simulink model "TosAeb"  (R2021a)
settings: Solver=FixedStepDiscrete  StartTime=0.0  StopTime=inf  FixedStep=0.01  RelTol=1e-3
undefined symbols (not defined in the file or in its folder): ACCModSts, AccSts1, AEB_ObjectMovingOrientation, AEB_ObjectMovingState, CN_BTCActive, CN_CurrentGearR, CN_CurrentGearR_DHT, CN_PTCActive, CN_VDCActive, CN_VSC_EspOff, CN_VSC_FCW_AEB_Error, CN_VSC_FCW_AEB_NoDispay, CN_VSC_FCW_AEB_PerfmDgdtn, CN_VSC_FCW_AEB_TempUnAvlbl, CN_VSC_VehStandstill, CNaccelAvoidMax, CNaccelAvoidMin, CNbrakePedalPress, CNcomfortStrLeftAccelMax, CNcomfortStrLeftAccelMin, CNcomfortStrRightAccelMax, CNcomfortStrRightAccelMin, CNDrvDoorOpen, CNDrvModeEco, CNDrvModeNorm, CNDrvModeOther, CNDrvModeSport, CNDrvSBRreminder, CNNull, CNobjInvalidID, CNPassDoorOpen, CNPassSBRreminder, CNPEBSAccelNegInf, CNPEBSAccelNull, CNPEBSTimeInf, CNPEBSTimeNull, CNSTOPMAX, CNSTOPNULL, CNsubjWidth, CNSwtOFF, CNSwtON, CNTTCMAX, CNTTCNULL, CNVehConfigAEBCycNone, CNVehConfigAEBNone, CNVehConfigAEBPedNone, CNVehConfigFCWNone, CNVehConfigJANone, CollisionSeverity, FALSE, FCWSnvty, JACycObjCalcInfoDefaultParams, JAPedObjCalcInfoDefaultParams, JAVehObjCalcInfoDefaultParams, latCycObjCalcInfoDefaultParams, latPedObjCalcInfoDefaultParams, longObjCalcInfoDefaultParams, ModSts, movingStatus, ObjectType ... (+1112)
libraries: simulink x3031, AEBSFunctionLib x50, Ta_Library x33, AEBSStateMachineLib x23, Mylibrary x12, simulink_extras x7, ObjSelect_lib x3, dspstat3 x2
(part 84 of 102)

== system TosAeb/FcwAebJa_Step_sys/ALG_AEBSystem/ALG_AEBController/AEB_LongTgtInfoCalc/LongObjCalcInfoCalc/AEBS_LongCollisionAssesApp/LongCollisionAvoidAssess/HighLvlBrkCalc/HighLvlBrkCalc4Warning/accelAvoidHighLvlBrkSituation1/accelAvoidLowLvlBrkCalc2/Situation1/Case1 ==
  axAbsObj : Inport
  vx : Inport  Port=2
  dxEgoBrake : Inport  Port=3
  Action Port : ActionPort
  Divide : Product  Inputs=*/
  Gain : Gain  Gain=2
  Math Function : Math  Operator=square  SignedPower=on  SaturateOnIntegerOverflow=on
  Subtract : Sum  Inputs=+-
  accelAvoid : Outport
  wires:
  vx/1 -> Math Function/1
  Math Function/1 -> Divide/1
  Gain/1 -> Divide/2
  dxEgoBrake/1 -> Gain/1
  Divide/1 -> Subtract/2
  axAbsObj/1 -> Subtract/1
  Subtract/1 -> accelAvoid/1

== system TosAeb/FcwAebJa_Step_sys/ALG_AEBSystem/ALG_AEBController/AEB_LongTgtInfoCalc/LongObjCalcInfoCalc/AEBS_LongCollisionAssesApp/LongCollisionAvoidAssess/HighLvlBrkCalc/HighLvlBrkCalc4Warning/accelAvoidHighLvlBrkSituation1/accelAvoidLowLvlBrkCalc2/Situation1/Case2 ==
  note: vEgo^2
      | aAvoid = ----------------------------------
      |                   vObj^2
      |                  ---------  - 2(dRel - dScure)
      |                   aObj
  subjVel : Inport
  vxAbsObj : Inport  Port=2
  axAbsObj : Inport  Port=3
  dxEgoBrake : Inport  Port=4
  Action Port : ActionPort
  Divide : Product  Inputs=*/
  Divide1 : Product  Inputs=*/
  Gain : Gain  Gain=2
  Math Function : Math  Operator=square  SignedPower=on  SaturateOnIntegerOverflow=on
  Math Function1 : Math  Operator=square  SignedPower=on  SaturateOnIntegerOverflow=on
  Subtract : Sum  Inputs=+-
  accelAvoid : Outport
  wires:
  vxAbsObj/1 -> Math Function/1
  Math Function/1 -> Divide/1
  axAbsObj/1 -> Divide/2
  Divide/1 -> Subtract/1
  dxEgoBrake/1 -> Gain/1
  Gain/1 -> Subtract/2
  subjVel/1 -> Math Function1/1
  Subtract/1 -> Divide1/2
  Math Function1/1 -> Divide1/1
  Divide1/1 -> accelAvoid/1

== system TosAeb/FcwAebJa_Step_sys/ALG_AEBSystem/ALG_AEBController/AEB_LongTgtInfoCalc/LongObjCalcInfoCalc/AEBS_LongCollisionAssesApp/LongCollisionAvoidAssess/HighLvlBrkCalc/HighLvlBrkCalc4Warning/accelAvoidHighLvlBrkSituation1/accelAvoidLowLvlBrkCalc2/Situation1/collisionFirstFlgCalc ==
  note: Calculate Ego and Obj Collision Time and
      |  Determine if the Collision Time is less than the object stop time
  dxEgoBrake : Inport
  vx : Inport  Port=2
  tObjStop : Inport  Port=3
  Divide : Product  Inputs=*/
  Gain : Gain  Gain=-1
  Gain1 : Gain  Gain=2
  Relational Operator : RelationalOperator  Operator=<  OutDataTypeStr=boolean  RndMeth=Simplest
  Saturation : Saturate  UpperLimit=CNTTCMAX  LowerLimit=CNTTCNULL
      settings: AttributesFormatString=|
      | UpperLimit:%<UpperLimit>
      | LowerLimit:%<LowerLimit>
  collisionFirstFlg : Outport
  wires:
  Divide/1 -> Gain1/1
  dxEgoBrake/1 -> Divide/1
  vx/1 -> Gain/1
  Gain/1 -> Divide/2
  Gain1/1 -> Saturation/1
  tObjStop/1 -> Relational Operator/2
  Relational Operator/1 -> collisionFirstFlg/1
  Saturation/1 -> Relational Operator/1

== system TosAeb/FcwAebJa_Step_sys/ALG_AEBSystem/ALG_AEBController/AEB_LongTgtInfoCalc/LongObjCalcInfoCalc/AEBS_LongCollisionAssesApp/LongCollisionAvoidAssess/HighLvlBrkCalc/HighLvlBrkCalc4Warning/accelAvoidHighLvlBrkSituation2/accelAvoidHighLvlBrkCalc/If Action Subsystem1/accelAvoidLowLvlBrkCalc ==
  vx : Inport
  axAbsObj : Inport  Port=2
  vxAbsObj : Inport  Port=3
  subjVel : Inport  Port=4
  tObjStop : Inport  Port=5
  dxEgoBrake : Inport  Port=6
  AccelAvoidSituationJudge : Stateflow chart  TreatAsAtomicUnit=on
  Bus Creator : BusCreator  Inputs=6
  Bus Selector : BusSelector  OutputSignals=vx,axAbsObj
  Bus Selector1 : BusSelector  OutputSignals=dxEgoBrake,vx,tObjStop,subjVel,vxAbsObj,axAbsObj
  Bus Selector2 : BusSelector  OutputSignals=axAbsObj,vx,dxEgoBrake
  Bus Selector3 : BusSelector  OutputSignals=subjVel,vxAbsObj,axAbsObj,dxEgoBrake
  Merge : Merge  Inputs=4
  Situation1 : Subsystem  TreatAsAtomicUnit=on
  Situation2 : Subsystem  TreatAsAtomicUnit=on
  Situation3 : Subsystem  TreatAsAtomicUnit=on
  Situation4 : Subsystem  TreatAsAtomicUnit=on
  accelAvoidLowLvlBrkLimit : Subsystem
  accelAvoidLowLvlBrk : Outport
  wires:
  accelAvoidLowLvlBrkLimit/1(accelAvoidLowLvlBrk) -> accelAvoidLowLvlBrk/1
  Merge/1 -> accelAvoidLowLvlBrkLimit/1(accelAvoidLowLvlBrkNoLimit)
  Bus Selector3/4 -> Situation3/4(dxEgoBrake)  "<dxEgoBrake>"
  Bus Selector3/3 -> Situation3/3(axAbsObj)  "<axAbsObj>"
  Bus Selector3/2 -> Situation3/2(vxAbsObj)  "<vxAbsObj>"
  Bus Selector3/1 -> Situation3/1(subjVel)  "<subjVel>"
  Bus Selector2/1 -> Situation2/1(axAbsObj)  "<axAbsObj>"
  Bus Selector2/2 -> Situation2/2(vx)  "<vx>"
  Bus Selector2/3 -> Situation2/3(dxEgoBrake)  "<dxEgoBrake>"
  Bus Selector/2 -> AccelAvoidSituationJudge/2(axAbsObj)  "<axAbsObj>"
  Bus Selector/1 -> AccelAvoidSituationJudge/1(vx)  "<vx>"
  Bus Creator/1 -> Bus Selector/1, Bus Selector1/1, Bus Selector2/1, Bus Selector3/1
  tObjStop/1 -> Bus Creator/5  "tObjStop"
  axAbsObj/1 -> Bus Creator/2  "axAbsObj"
  vx/1 -> Bus Creator/1  "vx"
  dxEgoBrake/1 -> Bus Creator/6  "dxEgoBrake"
  subjVel/1 -> Bus Creator/4  "subjVel"
  vxAbsObj/1 -> Bus Creator/3  "vxAbsObj"
  [Situation4] -> Situation4/Trigger
  [Situation3] -> Situation3/Trigger
  [Situation2] -> Situation2/Trigger
  AccelAvoidSituationJudge/4(Situation4()) -> [Situation4]
  AccelAvoidSituationJudge/3(Situation3()) -> [Situation3]
  AccelAvoidSituationJudge/2(Situation2()) -> [Situation2]
  Situation4/1(accelAvoidLowLvlBrk) -> Merge/4
  Situation3/1(accelAvoidLowLvlBrk) -> Merge/3
  Situation2/1(accelAvoidLowLvlBrk) -> Merge/2
  Situation1/1(accelAvoidLowLvlBrk) -> Merge/1
  AccelAvoidSituationJudge/1(Situation1()) -> Situation1/Trigger
  Bus Selector1/1 -> Situation1/1(dxEgoBrake)  "<dxEgoBrake>"
  Bus Selector1/2 -> Situation1/2(vx)  "<vx>"
  Bus Selector1/3 -> Situation1/3(tObjStop)  "<tObjStop>"
  Bus Selector1/4 -> Situation1/4(subjVel)  "<subjVel>"
  Bus Selector1/5 -> Situation1/5(vxAbsObj)  "<vxAbsObj>"
  Bus Selector1/6 -> Situation1/6(axAbsObj)  "<axAbsObj>"

== system TosAeb/FcwAebJa_Step_sys/ALG_AEBSystem/ALG_AEBController/AEB_LongTgtInfoCalc/LongObjCalcInfoCalc/AEBS_LongCollisionAssesApp/LongCollisionAvoidAssess/HighLvlBrkCalc/HighLvlBrkCalc4Warning/accelAvoidHighLvlBrkSituation2/accelAvoidHighLvlBrkCalc/If Action Subsystem1/accelAvoidLowLvlBrkCalc1 ==
  vx : Inport
  axAbsObj : Inport  Port=2
  vxAbsObj : Inport  Port=3
  subjVel : Inport  Port=4
  tObjStop : Inport  Port=5
  dxEgoBrake : Inport  Port=6
  AccelAvoidSituationJudge : Stateflow chart  TreatAsAtomicUnit=on
  Bus Creator : BusCreator  Inputs=6
  Bus Selector : BusSelector  OutputSignals=vxRelTargetObjCalc,axAbsTargetObjCalc
  Bus Selector1 : BusSelector  OutputSignals=dxEgoBrake,vxRelTargetObjCalc,tObjStop,subjVel,vxAbsTargetObjCalc,axAbsTargetObjCalc
  Bus Selector2 : BusSelector  OutputSignals=axAbsTargetObjCalc,vxRelTargetObjCalc,dxEgoBrake
  Bus Selector3 : BusSelector  OutputSignals=subjVel,vxAbsTargetObjCalc,axAbsTargetObjCalc,dxEgoBrake
  Merge : Merge  Inputs=4
  Situation1 : Subsystem  TreatAsAtomicUnit=on
  Situation2 : Subsystem  TreatAsAtomicUnit=on
  Situation3 : Subsystem  TreatAsAtomicUnit=on
  Situation4 : Subsystem  TreatAsAtomicUnit=on
  accelAvoidLowLvlBrkLimit : Subsystem
  accelAvoidLowLvlBrk : Outport
  wires:
  Bus Selector1/6 -> Situation1/6(axAbsObj)  "<axAbsTargetObjCalc>"
  Bus Selector1/5 -> Situation1/5(vxAbsObj)  "<vxAbsTargetObjCalc>"
  Bus Selector1/4 -> Situation1/4(subjVel)  "<subjVel>"
  Bus Selector1/3 -> Situation1/3(tObjStop)  "<tObjStop>"
  Bus Selector1/2 -> Situation1/2(vx)  "<vxRelTargetObjCalc>"
  Bus Selector1/1 -> Situation1/1(dxEgoBrake)  "<dxEgoBrake>"
  AccelAvoidSituationJudge/1(Situation1()) -> Situation1/Trigger
  Situation1/1(accelAvoidLowLvlBrk) -> Merge/1
  Situation2/1(accelAvoidLowLvlBrk) -> Merge/2
  Situation3/1(accelAvoidLowLvlBrk) -> Merge/3
  Situation4/1(accelAvoidLowLvlBrk) -> Merge/4
  AccelAvoidSituationJudge/2(Situation2()) -> [Situation2]
  AccelAvoidSituationJudge/3(Situation3()) -> [Situation3]
  AccelAvoidSituationJudge/4(Situation4()) -> [Situation4]
  [Situation2] -> Situation2/Trigger
  [Situation3] -> Situation3/Trigger
  [Situation4] -> Situation4/Trigger
  vxAbsObj/1 -> Bus Creator/3  "vxAbsTargetObjCalc"
  subjVel/1 -> Bus Creator/4  "subjVel"
  dxEgoBrake/1 -> Bus Creator/6  "dxEgoBrake"
  vx/1 -> Bus Creator/1  "vxRelTargetObjCalc"
  axAbsObj/1 -> Bus Creator/2  "axAbsTargetObjCalc"
  tObjStop/1 -> Bus Creator/5  "tObjStop"
  Bus Creator/1 -> Bus Selector3/1, Bus Selector2/1, Bus Selector1/1, Bus Selector/1
  Bus Selector/1 -> AccelAvoidSituationJudge/1(vxRelTargetObjCalc)  "<vxRelTargetObjCalc>"
  Bus Selector/2 -> AccelAvoidSituationJudge/2(axAbsTargetObjCalc)  "<axAbsTargetObjCalc>"
  Bus Selector2/3 -> Situation2/3(dxEgoBrake)  "<dxEgoBrake>"
  Bus Selector2/2 -> Situation2/2(vx)  "<vxRelTargetObjCalc>"
  Bus Selector2/1 -> Situation2/1(axAbsObj)  "<axAbsTargetObjCalc>"
  Bus Selector3/1 -> Situation3/1(subjVel)  "<subjVel>"
  Bus Selector3/2 -> Situation3/2(vxAbsObj)  "<vxAbsTargetObjCalc>"
  Bus Selector3/3 -> Situation3/3(axAbsObj)  "<axAbsTargetObjCalc>"
  Bus Selector3/4 -> Situation3/4(dxEgoBrake)  "<dxEgoBrake>"
  Merge/1 -> accelAvoidLowLvlBrkLimit/1(accelAvoidLowLvlBrkNoLimit)
  accelAvoidLowLvlBrkLimit/1(accelAvoidLowLvlBrk) -> accelAvoidLowLvlBrk/1

== system TosAeb/FcwAebJa_Step_sys/ALG_AEBSystem/ALG_AEBController/AEB_LongTgtInfoCalc/LongObjCalcInfoCalc/AEBS_LongCollisionAssesApp/LongCollisionAvoidAssess/HighLvlBrkCalc/HighLvlBrkCalc4Warning/accelAvoidHighLvlBrkSituation2/accelAvoidHighLvlBrkCalc/tObjStopCalc/StopTimeCalc ==
  velRel : Inport
  accelRel : Inport  Port=2
  tStopFuncCalc : TriggerPort  TriggerType=function-call  VariantControl=Variant
  Abs : Abs
  Abs1 : Abs
  Divide : Product  Inputs=*/
  tStop : Outport
  wires:
  velRel/1 -> Abs/1
  accelRel/1 -> Abs1/1
  Abs/1 -> Divide/1
  Abs1/1 -> Divide/2
  Divide/1 -> tStop/1

== system TosAeb/FcwAebJa_Step_sys/ALG_AEBSystem/ALG_AEBController/AEB_LongTgtInfoCalc/LongObjCalcInfoCalc/AEBS_LongCollisionAssesApp/LongCollisionAvoidAssess/HighLvlBrkCalc/HighLvlBrkCalc4Warning/accelAvoidHighLvlBrkSituation3/If Action Subsystem1/DistReactionCalc/If Action Subsystem ==
  Action Port : ActionPort  ActionPortLabel=if(u1)
  Constant : Constant  Value=CNNull
  dxObjReaction : Outport
  wires:
  Constant/1 -> dxObjReaction/1

== system TosAeb/FcwAebJa_Step_sys/ALG_AEBSystem/ALG_AEBController/AEB_LongTgtInfoCalc/LongObjCalcInfoCalc/AEBS_LongCollisionAssesApp/LongCollisionAvoidAssess/HighLvlBrkCalc/HighLvlBrkCalc4Warning/accelAvoidHighLvlBrkSituation3/If Action Subsystem1/DistReactionCalc/If Action Subsystem1 ==
  vxAbsObj : Inport
  axAbsObj : Inport  Port=2
  Action Port : ActionPort  ActionPortLabel=else
  Divide : Product  Inputs=*/
  Gain : Gain  Gain=-2
  Math Function : Math  Operator=square  SignedPower=on  SaturateOnIntegerOverflow=on
  dxObjReaction : Outport
  wires:
  Divide/1 -> dxObjReaction/1
  Gain/1 -> Divide/2
  axAbsObj/1 -> Gain/1
  Math Function/1 -> Divide/1
  vxAbsObj/1 -> Math Function/1

== system TosAeb/FcwAebJa_Step_sys/ALG_AEBSystem/ALG_AEBController/AEB_LongTgtInfoCalc/LongObjCalcInfoCalc/AEBS_LongCollisionAssesApp/LongCollisionAvoidAssess/HighLvlBrkCalc/HighLvlBrkCalc4Warning/accelAvoidHighLvlBrkSituation3/If Action Subsystem1/accelAvoidHighLvlBrkCalc/MovingSateCalcAfterReactionTime ==
  note: For Situation 3,after driver reaction time ,the object has been stopped.
      | Ego vehicle is keeping moving.
  subjLongAccel : Inport
  tReaction : Inport  Port=2
  subjVel : Inport  Port=3
  Add2 : Sum
  Constant : Constant  Value=CNNull
  Product3 : Product
  Subtract : Sum  Inputs=+-
  vxRelTargetPredict : Outport
  axAbsTargetPredict : Outport  Port=2
  vxAbsTargetPredict : Outport  Port=3
  subjVelPredict : Outport  Port=4
  tObjStopPredict : Outport  Port=5
  wires:
  Add2/1 -> subjVelPredict/1, Subtract/2
  Subtract/1 -> vxRelTargetPredict/1
  Constant/1 -> axAbsTargetPredict/1, tObjStopPredict/1, vxAbsTargetPredict/1, Subtract/1
  tReaction/1 -> Product3/2
  subjLongAccel/1 -> Product3/1
  Product3/1 -> Add2/1
  subjVel/1 -> Add2/2

== system TosAeb/FcwAebJa_Step_sys/ALG_AEBSystem/ALG_AEBController/AEB_LongTgtInfoCalc/LongObjCalcInfoCalc/AEBS_LongCollisionAssesApp/LongCollisionAvoidAssess/HighLvlBrkCalc/HighLvlBrkCalc4Warning/accelAvoidHighLvlBrkSituation3/If Action Subsystem1/accelAvoidHighLvlBrkCalc/accelAvoidLowLvlBrkCalc ==
  vx : Inport
  axAbsObj : Inport  Port=2
  vxAbsObj : Inport  Port=3
  subjVel : Inport  Port=4
  tObjStop : Inport  Port=5
  dxEgoBrake : Inport  Port=6
  AccelAvoidSituationJudge : Stateflow chart  TreatAsAtomicUnit=on
  Bus Creator : BusCreator  Inputs=6
  Bus Selector : BusSelector  OutputSignals=vx,axAbsObj
  Bus Selector1 : BusSelector  OutputSignals=dxEgoBrake,vx,tObjStop,subjVel,vxAbsObj,axAbsObj
  Bus Selector2 : BusSelector  OutputSignals=axAbsObj,vx,dxEgoBrake
  Bus Selector3 : BusSelector  OutputSignals=subjVel,vxAbsObj,axAbsObj,dxEgoBrake
  Merge : Merge  Inputs=4
  Situation1 : Subsystem  TreatAsAtomicUnit=on
  Situation2 : Subsystem  TreatAsAtomicUnit=on
  Situation3 : Subsystem  TreatAsAtomicUnit=on
  Situation4 : Subsystem  TreatAsAtomicUnit=on
  accelAvoidLowLvlBrkLimit : Subsystem
  accelAvoidLowLvlBrk : Outport
  wires:
  accelAvoidLowLvlBrkLimit/1(accelAvoidLowLvlBrk) -> accelAvoidLowLvlBrk/1
  Merge/1 -> accelAvoidLowLvlBrkLimit/1(accelAvoidLowLvlBrkNoLimit)
  Bus Selector3/4 -> Situation3/4(dxEgoBrake)  "<dxEgoBrake>"
  Bus Selector3/3 -> Situation3/3(axAbsObj)  "<axAbsObj>"
  Bus Selector3/2 -> Situation3/2(vxAbsObj)  "<vxAbsObj>"
  Bus Selector3/1 -> Situation3/1(subjVel)  "<subjVel>"
  Bus Selector2/1 -> Situation2/1(axAbsObj)  "<axAbsObj>"
  Bus Selector2/2 -> Situation2/2(vx)  "<vx>"
  Bus Selector2/3 -> Situation2/3(dxEgoBrake)  "<dxEgoBrake>"
  Bus Selector/2 -> AccelAvoidSituationJudge/2(axAbsObj)  "<axAbsObj>"
  Bus Selector/1 -> AccelAvoidSituationJudge/1(vx)  "<vx>"
  Bus Creator/1 -> Bus Selector/1, Bus Selector1/1, Bus Selector2/1, Bus Selector3/1
  tObjStop/1 -> Bus Creator/5  "tObjStop"
  axAbsObj/1 -> Bus Creator/2  "axAbsObj"
  vx/1 -> Bus Creator/1  "vx"
  dxEgoBrake/1 -> Bus Creator/6  "dxEgoBrake"
  subjVel/1 -> Bus Creator/4  "subjVel"
  vxAbsObj/1 -> Bus Creator/3  "vxAbsObj"
  [Situation4] -> Situation4/Trigger
  [Situation3] -> Situation3/Trigger
  [Situation2] -> Situation2/Trigger
  AccelAvoidSituationJudge/4(Situation4()) -> [Situation4]
  AccelAvoidSituationJudge/3(Situation3()) -> [Situation3]
  AccelAvoidSituationJudge/2(Situation2()) -> [Situation2]
  Situation4/1(accelAvoidLowLvlBrk) -> Merge/4
  Situation3/1(accelAvoidLowLvlBrk) -> Merge/3
  Situation2/1(accelAvoidLowLvlBrk) -> Merge/2
  Situation1/1(accelAvoidLowLvlBrk) -> Merge/1
  AccelAvoidSituationJudge/1(Situation1()) -> Situation1/Trigger
  Bus Selector1/1 -> Situation1/1(dxEgoBrake)  "<dxEgoBrake>"
  Bus Selector1/2 -> Situation1/2(vx)  "<vx>"
  Bus Selector1/3 -> Situation1/3(tObjStop)  "<tObjStop>"
  Bus Selector1/4 -> Situation1/4(subjVel)  "<subjVel>"
  Bus Selector1/5 -> Situation1/5(vxAbsObj)  "<vxAbsObj>"
  Bus Selector1/6 -> Situation1/6(axAbsObj)  "<axAbsObj>"

== system TosAeb/FcwAebJa_Step_sys/ALG_AEBSystem/ALG_AEBController/AEB_LongTgtInfoCalc/LongObjCalcInfoCalc/AEBS_LongCollisionAssesApp/LongCollisionAvoidAssess/HighLvlBrkCalc/HighLvlBrkCalc4Warning/accelAvoidHighLvlBrkSituation3/If Action Subsystem1/accelAvoidHighLvlBrkCalc/accelAvoidLowLvlBrkCalc1 ==
  vx : Inport
  axAbsObj : Inport  Port=2
  vxAbsObj : Inport  Port=3
  subjVel : Inport  Port=4
  tObjStop : Inport  Port=5
  dxEgoBrake : Inport  Port=6
  AccelAvoidSituationJudge : Stateflow chart  TreatAsAtomicUnit=on
  Bus Creator : BusCreator  Inputs=6
  Bus Selector : BusSelector  OutputSignals=vxRelTargetObjCalc,axAbsTargetObjCalc
  Bus Selector1 : BusSelector  OutputSignals=dxEgoBrake,vxRelTargetObjCalc,tObjStop,subjVel,vxAbsTargetObjCalc,axAbsTargetObjCalc
  Bus Selector2 : BusSelector  OutputSignals=axAbsTargetObjCalc,vxRelTargetObjCalc,dxEgoBrake
  Bus Selector3 : BusSelector  OutputSignals=subjVel,vxAbsTargetObjCalc,axAbsTargetObjCalc,dxEgoBrake
  Merge : Merge  Inputs=4
  Situation1 : Subsystem  TreatAsAtomicUnit=on
  Situation2 : Subsystem  TreatAsAtomicUnit=on
  Situation3 : Subsystem  TreatAsAtomicUnit=on
  Situation4 : Subsystem  TreatAsAtomicUnit=on
  accelAvoidLowLvlBrkLimit : Subsystem
  accelAvoidLowLvlBrk : Outport
  wires:
  Bus Selector1/6 -> Situation1/6(axAbsObj)  "<axAbsTargetObjCalc>"
  Bus Selector1/5 -> Situation1/5(vxAbsObj)  "<vxAbsTargetObjCalc>"
  Bus Selector1/4 -> Situation1/4(subjVel)  "<subjVel>"
  Bus Selector1/3 -> Situation1/3(tObjStop)  "<tObjStop>"
  Bus Selector1/2 -> Situation1/2(vx)  "<vxRelTargetObjCalc>"
  Bus Selector1/1 -> Situation1/1(dxEgoBrake)  "<dxEgoBrake>"
  AccelAvoidSituationJudge/1(Situation1()) -> Situation1/Trigger
  Situation1/1(accelAvoidLowLvlBrk) -> Merge/1
  Situation2/1(accelAvoidLowLvlBrk) -> Merge/2
  Situation3/1(accelAvoidLowLvlBrk) -> Merge/3
  Situation4/1(accelAvoidLowLvlBrk) -> Merge/4
  AccelAvoidSituationJudge/2(Situation2()) -> [Situation2]
  AccelAvoidSituationJudge/3(Situation3()) -> [Situation3]
  AccelAvoidSituationJudge/4(Situation4()) -> [Situation4]
  [Situation2] -> Situation2/Trigger
  [Situation3] -> Situation3/Trigger
  [Situation4] -> Situation4/Trigger
  vxAbsObj/1 -> Bus Creator/3  "vxAbsTargetObjCalc"
  subjVel/1 -> Bus Creator/4  "subjVel"
  dxEgoBrake/1 -> Bus Creator/6  "dxEgoBrake"
  vx/1 -> Bus Creator/1  "vxRelTargetObjCalc"
  axAbsObj/1 -> Bus Creator/2  "axAbsTargetObjCalc"
  tObjStop/1 -> Bus Creator/5  "tObjStop"
  Bus Creator/1 -> Bus Selector3/1, Bus Selector2/1, Bus Selector1/1, Bus Selector/1
  Bus Selector/1 -> AccelAvoidSituationJudge/1(vxRelTargetObjCalc)  "<vxRelTargetObjCalc>"
  Bus Selector/2 -> AccelAvoidSituationJudge/2(axAbsTargetObjCalc)  "<axAbsTargetObjCalc>"
  Bus Selector2/3 -> Situation2/3(dxEgoBrake)  "<dxEgoBrake>"
  Bus Selector2/2 -> Situation2/2(vx)  "<vxRelTargetObjCalc>"
  Bus Selector2/1 -> Situation2/1(axAbsObj)  "<axAbsTargetObjCalc>"
  Bus Selector3/1 -> Situation3/1(subjVel)  "<subjVel>"
  Bus Selector3/2 -> Situation3/2(vxAbsObj)  "<vxAbsTargetObjCalc>"
  Bus Selector3/3 -> Situation3/3(axAbsObj)  "<axAbsTargetObjCalc>"
  Bus Selector3/4 -> Situation3/4(dxEgoBrake)  "<dxEgoBrake>"
  Merge/1 -> accelAvoidLowLvlBrkLimit/1(accelAvoidLowLvlBrkNoLimit)
  accelAvoidLowLvlBrkLimit/1(accelAvoidLowLvlBrk) -> accelAvoidLowLvlBrk/1

== system TosAeb/FcwAebJa_Step_sys/ALG_AEBSystem/ALG_AEBController/AEB_LongTgtInfoCalc/LongObjCalcInfoCalc/AEBS_LongCollisionAssesApp/LongCollisionAvoidAssess/HighLvlBrkCalc/HighLvlBrkCalc4Warning/accelAvoidHighLvlBrkSituation5/If Action Subsystem1/If Action Subsystem1/If Action Subsystem2 ==
  Action Port : ActionPort  ActionPortLabel=if(u1)
  Constant : Constant  Value=CNNull
  dxObjReaction : Outport
  wires:
  Constant/1 -> dxObjReaction/1

== system TosAeb/FcwAebJa_Step_sys/ALG_AEBSystem/ALG_AEBController/AEB_LongTgtInfoCalc/LongObjCalcInfoCalc/AEBS_LongCollisionAssesApp/LongCollisionAvoidAssess/HighLvlBrkCalc/HighLvlBrkCalc4Warning/accelAvoidHighLvlBrkSituation5/If Action Subsystem1/If Action Subsystem1/If Action Subsystem3 ==
  vxAbsObj : Inport
  axAbsObj : Inport  Port=2
  Action Port : ActionPort  ActionPortLabel=else
  Divide : Product  Inputs=*/
  Gain : Gain  Gain=-2
  Math Function : Math  Operator=square  SignedPower=on  SaturateOnIntegerOverflow=on
  dxObjReaction : Outport
  wires:
  Divide/1 -> dxObjReaction/1
  Gain/1 -> Divide/2
  axAbsObj/1 -> Gain/1
  Math Function/1 -> Divide/1
  vxAbsObj/1 -> Math Function/1

== system TosAeb/FcwAebJa_Step_sys/ALG_AEBSystem/ALG_AEBController/AEB_LongTgtInfoCalc/LongObjCalcInfoCalc/AEBS_LongCollisionAssesApp/collisionSeverityHighCalc/collisionSeverityHighCalc1/CollisionAssessHighCalcVersion3/CollisionAssessHighCalc1/CalcBrakePhase1/tEgoStopCalc/tStopCalc ==
  accelAbs : Inport
  velAbs : Inport  Port=2
  StopTimeFlow : Stateflow chart  TreatAsAtomicUnit=on
  tStop : Outport
  wires:
  velAbs/1 -> StopTimeFlow/2(velRel)  "velAbs"
  accelAbs/1 -> StopTimeFlow/1(accelRel)  "accelAbs"
  StopTimeFlow/1(tStop) -> tStop/1  "tStop"

== system TosAeb/FcwAebJa_Step_sys/ALG_AEBSystem/ALG_AEBController/AEB_LongTgtInfoCalc/LongObjCalcInfoCalc/AEBS_LongCollisionAssesApp/collisionSeverityHighCalc/collisionSeverityHighCalc1/CollisionAssessHighCalcVersion3/CollisionAssessHighCalc1/CalcBrakePhase1/tObjStopCalc/tStopCalc ==
  accelAbs : Inport
  velAbs : Inport  Port=2
  StopTimeFlow : Stateflow chart  TreatAsAtomicUnit=on
  tStop : Outport
  wires:
  velAbs/1 -> StopTimeFlow/2(velRel)  "velAbs"
  accelAbs/1 -> StopTimeFlow/1(accelRel)  "accelAbs"
  StopTimeFlow/1(tStop) -> tStop/1  "tStop"

== system TosAeb/FcwAebJa_Step_sys/ALG_AEBSystem/ALG_AEBController/AEB_LongTgtInfoCalc/LongObjCalcInfoCalc/AEBS_LongCollisionAssesApp/collisionSeverityHighCalc/collisionSeverityHighCalc1/CollisionAssessHighCalcVersion3/CollisionAssessHighCalc1/CalcBrakePhase1/timeEgovDeltaCalc/timeEgovDelata ==
  accelAbs : Inport
  velAbs : Inport  Port=2
  StopTimeFlow : Stateflow chart  TreatAsAtomicUnit=on
  tStop : Outport
  wires:
  velAbs/1 -> StopTimeFlow/2(velRel)  "velAbs"
  accelAbs/1 -> StopTimeFlow/1(accelRel)  "accelAbs"
  StopTimeFlow/1(tStop) -> tStop/1  "tStop"

== system TosAeb/FcwAebJa_Step_sys/ALG_AEBSystem/ALG_AEBController/AEB_LongTgtInfoCalc/LongObjCalcInfoCalc/AEBS_LongCollisionAssesApp/collisionSeverityHighCalc/collisionSeverityHighCalc1/CollisionAssessHighCalcVersion3/CollisionAssessHighCalc1/CalcBrakePhase1/timeEgovDeltaCalc/velMaxReudceFiltCalc ==
  v0Ego : Inport
  velMaxReudce : Inport  Port=2
  MinMax : MinMax  Inputs=2
  velMaxReudceFilt : Outport
  wires:
  MinMax/1 -> velMaxReudceFilt/1
  velMaxReudce/1 -> MinMax/2
  v0Ego/1 -> MinMax/1

== system TosAeb/FcwAebJa_Step_sys/ALG_AEBSystem/ALG_AEBController/AEB_LongTgtInfoCalc/LongObjCalcInfoCalc/AEBS_LongCollisionAssesApp/collisionSeverityHighCalc/collisionSeverityHighCalc1/CollisionAssessHighCalcVersion3/CollisionAssessHighCalc1/CalcBrakePhase2/BrkSysRuntimeFhase2/If Action Subsystem ==
  a0Ego : Inport
  accelMaxDec : Inport  Port=2
  accelGradient : Inport  Port=3
  Action Port : ActionPort  ActionPortLabel=if(u1)
  Divide : Product  Inputs=*/
  Subtract : Sum  Inputs=+-
  tTime : Outport
  wires:
  accelMaxDec/1 -> Subtract/1
  a0Ego/1 -> Subtract/2
  Subtract/1 -> Divide/1
  accelGradient/1 -> Divide/2
  Divide/1 -> tTime/1

== system TosAeb/FcwAebJa_Step_sys/ALG_AEBSystem/ALG_AEBController/AEB_LongTgtInfoCalc/LongObjCalcInfoCalc/AEBS_LongCollisionAssesApp/collisionSeverityHighCalc/collisionSeverityHighCalc1/CollisionAssessHighCalcVersion3/CollisionAssessHighCalc1/CalcBrakePhase2/BrkSysRuntimeFhase2/If Action Subsystem1 ==
  Action Port : ActionPort  ActionPortLabel=else
  Constant : Constant  Value=CNNull
  tTime : Outport
  wires:
  Constant/1 -> tTime/1

== system TosAeb/FcwAebJa_Step_sys/ALG_AEBSystem/ALG_AEBController/AEB_LongTgtInfoCalc/LongObjCalcInfoCalc/AEBS_LongCollisionAssesApp/collisionSeverityHighCalc/collisionSeverityHighCalc1/CollisionAssessHighCalcVersion3/CollisionAssessHighCalc1/CalcBrakePhase2/BrkSysRuntimeFhase2/accelEgoLessAccelmaxReduce ==
  accelMaxDec : Inport
  a0Ego : Inport  Port=2
  Relational Operator : RelationalOperator  Operator=<  OutDataTypeStr=boolean  RndMeth=Simplest
  Flg : Outport
  wires:
  Relational Operator/1 -> Flg/1
  accelMaxDec/1 -> Relational Operator/1
  a0Ego/1 -> Relational Operator/2

== system TosAeb/FcwAebJa_Step_sys/ALG_AEBSystem/ALG_AEBController/AEB_LongTgtInfoCalc/LongObjCalcInfoCalc/AEBS_LongCollisionAssesApp/collisionSeverityHighCalc/collisionSeverityHighCalc1/CollisionAssessHighCalcVersion3/CollisionAssessHighCalc1/CalcBrakePhase2/tEgoStopCalc/tStopCalc ==
  accelAbs : Inport
  velAbs : Inport  Port=2
  StopTimeFlow : Stateflow chart  TreatAsAtomicUnit=on
  tStop : Outport
  wires:
  velAbs/1 -> StopTimeFlow/2(velRel)  "velAbs"
  accelAbs/1 -> StopTimeFlow/1(accelRel)  "accelAbs"
  StopTimeFlow/1(tStop) -> tStop/1  "tStop"

== system TosAeb/FcwAebJa_Step_sys/ALG_AEBSystem/ALG_AEBController/AEB_LongTgtInfoCalc/LongObjCalcInfoCalc/AEBS_LongCollisionAssesApp/collisionSeverityHighCalc/collisionSeverityHighCalc1/CollisionAssessHighCalcVersion3/CollisionAssessHighCalc1/CalcBrakePhase2/tObjStopCalc/tStopCalc ==
  accelAbs : Inport
  velAbs : Inport  Port=2
  StopTimeFlow : Stateflow chart  TreatAsAtomicUnit=on
  tStop : Outport
  wires:
  velAbs/1 -> StopTimeFlow/2(velRel)  "velAbs"
  accelAbs/1 -> StopTimeFlow/1(accelRel)  "accelAbs"
  StopTimeFlow/1(tStop) -> tStop/1  "tStop"

== system TosAeb/FcwAebJa_Step_sys/ALG_AEBSystem/ALG_AEBController/AEB_LongTgtInfoCalc/LongObjCalcInfoCalc/AEBS_LongCollisionAssesApp/collisionSeverityHighCalc/collisionSeverityHighCalc1/CollisionAssessHighCalcVersion3/CollisionAssessHighCalc1/CalcBrakePhase2/timeEgovDeltaCalc/timeEgovDelata ==
  accelAbs : Inport
  velAbs : Inport  Port=2
  StopTimeFlow : Stateflow chart  TreatAsAtomicUnit=on
  tStop : Outport
  wires:
  velAbs/1 -> StopTimeFlow/2(velRel)  "velAbs"
  accelAbs/1 -> StopTimeFlow/1(accelRel)  "accelAbs"
  StopTimeFlow/1(tStop) -> tStop/1  "tStop"

== system TosAeb/FcwAebJa_Step_sys/ALG_AEBSystem/ALG_AEBController/AEB_LongTgtInfoCalc/LongObjCalcInfoCalc/AEBS_LongCollisionAssesApp/collisionSeverityHighCalc/collisionSeverityHighCalc1/CollisionAssessHighCalcVersion3/CollisionAssessHighCalc1/CalcBrakePhase2/timeEgovDeltaCalc/velMaxReudceFiltCalc ==
  v0Ego : Inport
  velMaxReudce : Inport  Port=2
  MinMax : MinMax  Inputs=2
  velMaxReudceFilt : Outport
  wires:
  MinMax/1 -> velMaxReudceFilt/1
  velMaxReudce/1 -> MinMax/2
  v0Ego/1 -> MinMax/1

== system TosAeb/FcwAebJa_Step_sys/ALG_AEBSystem/ALG_AEBController/AEB_LongTgtInfoCalc/LongObjCalcInfoCalc/AEBS_LongCollisionAssesApp/collisionSeverityHighCalc/collisionSeverityHighCalc1/CollisionAssessHighCalcVersion3/CollisionAssessHighCalc1/CalcBrakePhase3/BrkSysRuntime/Subsystem ==
  a0Obj : Inport
  accelMaxDec : Inport  Port=2
  Abs : Abs
  Compare To Constant : simulink/Logic and Bit Operations/Compare To Constant  relop=<  const=1e-6  OutDataTypeStr=boolean
  Subtract : Sum  Inputs=+-
  Flg : Outport
  wires:
  Abs/1 -> Compare To Constant/1
  accelMaxDec/1 -> Subtract/2
  a0Obj/1 -> Subtract/1
  Subtract/1 -> Abs/1
  Compare To Constant/1 -> Flg/1

== system TosAeb/FcwAebJa_Step_sys/ALG_AEBSystem/ALG_AEBController/AEB_LongTgtInfoCalc/LongObjCalcInfoCalc/AEBS_LongCollisionAssesApp/collisionSeverityHighCalc/collisionSeverityHighCalc1/CollisionAssessHighCalcVersion3/CollisionAssessHighCalc1/CalcBrakePhase3/BrkSysRuntime/a0ObjGreatSituation ==
  vtEgo : Inport
  accelMaxDec : Inport  Port=2
  v0Obj : Inport  Port=3
  a0Obj : Inport  Port=4
  Action Port : ActionPort  ActionPortLabel=else
  If : If  IfExpression=u1
  Merge : Merge
  Subsystem : Subsystem
  vtEgoGreatSituation : Subsystem  TreatAsAtomicUnit=on
  vtEgoLessSituation : Subsystem  TreatAsAtomicUnit=on
  tTime : Outport
  wires:
  [vtEgoGreat] -> vtEgoGreatSituation/Action
  If/2 -> [vtEgoGreat]
  Subsystem/1(Flg) -> If/1
  vtEgo/1 -> vtEgoLessSituation/2(vtEgo), Subsystem/1(vtEgo)
  v0Obj/1 -> vtEgoLessSituation/1(v0Obj), Subsystem/2(v0Obj)
  If/1 -> vtEgoLessSituation/Action
  vtEgoLessSituation/1(tTime) -> Merge/1
  vtEgoGreatSituation/1(tTime) -> Merge/2
  Merge/1 -> tTime/1
  accelMaxDec/1 -> vtEgoLessSituation/3(accelMaxDec)
  a0Obj/1 -> vtEgoLessSituation/4(a0Obj)

== system TosAeb/FcwAebJa_Step_sys/ALG_AEBSystem/ALG_AEBController/AEB_LongTgtInfoCalc/LongObjCalcInfoCalc/AEBS_LongCollisionAssesApp/collisionSeverityHighCalc/collisionSeverityHighCalc1/CollisionAssessHighCalcVersion3/CollisionAssessHighCalc1/CalcBrakePhase3/BrkSysRuntime/a0ObjLessSituation ==
  vtEgo : Inport
  accelMaxDec : Inport  Port=2
  Action Port : ActionPort  ActionPortLabel=if(u1)
  Divide : Product  Inputs=*/
  Unary Minus : UnaryMinus
  tTime : Outport
  wires:
  vtEgo/1 -> Unary Minus/1
  Unary Minus/1 -> Divide/1
  accelMaxDec/1 -> Divide/2
  Divide/1 -> tTime/1

== system TosAeb/FcwAebJa_Step_sys/ALG_AEBSystem/ALG_AEBController/AEB_LongTgtInfoCalc/LongObjCalcInfoCalc/AEBS_LongCollisionAssesApp/collisionSeverityHighCalc/collisionSeverityHighCalc1/CollisionAssessHighCalcVersion3/CollisionAssessHighCalc1/CalcBrakePhase3/tEgoStopCalc/tStopCalc ==
  accelAbs : Inport
  velAbs : Inport  Port=2
  StopTimeFlow : Stateflow chart  TreatAsAtomicUnit=on
  tStop : Outport
  wires:
  velAbs/1 -> StopTimeFlow/2(velRel)  "velAbs"
  accelAbs/1 -> StopTimeFlow/1(accelRel)  "accelAbs"
  StopTimeFlow/1(tStop) -> tStop/1  "tStop"

== system TosAeb/FcwAebJa_Step_sys/ALG_AEBSystem/ALG_AEBController/AEB_LongTgtInfoCalc/LongObjCalcInfoCalc/AEBS_LongCollisionAssesApp/collisionSeverityHighCalc/collisionSeverityHighCalc1/CollisionAssessHighCalcVersion3/CollisionAssessHighCalc1/CalcBrakePhase3/tObjStopCalc/tStopCalc ==
  accelAbs : Inport
  velAbs : Inport  Port=2
  StopTimeFlow : Stateflow chart  TreatAsAtomicUnit=on
  tStop : Outport
  wires:
  velAbs/1 -> StopTimeFlow/2(velRel)  "velAbs"
  accelAbs/1 -> StopTimeFlow/1(accelRel)  "accelAbs"
  StopTimeFlow/1(tStop) -> tStop/1  "tStop"

== system TosAeb/FcwAebJa_Step_sys/ALG_AEBSystem/ALG_AEBController/AEB_LongTgtInfoCalc/LongObjCalcInfoCalc/AEBS_LongCollisionAssesApp/collisionSeverityHighCalc/collisionSeverityHighCalc1/CollisionAssessHighCalcVersion3/CollisionAssessHighCalc1/CalcBrakePhase3/timeEgovDeltaCalc/timeEgovDelata ==
  accelAbs : Inport
  velAbs : Inport  Port=2
  StopTimeFlow : Stateflow chart  TreatAsAtomicUnit=on
  tStop : Outport
  wires:
  velAbs/1 -> StopTimeFlow/2(velRel)  "velAbs"
  accelAbs/1 -> StopTimeFlow/1(accelRel)  "accelAbs"
  StopTimeFlow/1(tStop) -> tStop/1  "tStop"

== system TosAeb/FcwAebJa_Step_sys/ALG_AEBSystem/ALG_AEBController/AEB_LongTgtInfoCalc/LongObjCalcInfoCalc/AEBS_LongCollisionAssesApp/collisionSeverityHighCalc/collisionSeverityHighCalc1/CollisionAssessHighCalcVersion3/CollisionAssessHighCalc1/CalcBrakePhase3/timeEgovDeltaCalc/velMaxReudceFiltCalc ==
  v0Ego : Inport
  velMaxReudce : Inport  Port=2
  MinMax : MinMax  Inputs=2
  velMaxReudceFilt : Outport
  wires:
  MinMax/1 -> velMaxReudceFilt/1
  velMaxReudce/1 -> MinMax/2
  v0Ego/1 -> MinMax/1

== system TosAeb/FcwAebJa_Step_sys/ALG_AEBSystem/ALG_AEBController/AEB_LongTgtInfoCalc/LongObjCalcInfoCalc/AEBS_LongCollisionAssesApp/collisionSeverityHighCalc/collisionSeverityHighCalc1/CollisionAssessHighCalcVersion3/CollisionAssessHighCalc2/CalcBrakePhase1/tEgoStopCalc/tStopCalc ==
  accelAbs : Inport
  velAbs : Inport  Port=2
  StopTimeFlow : Stateflow chart  TreatAsAtomicUnit=on
  tStop : Outport
  wires:
  velAbs/1 -> StopTimeFlow/2(velRel)  "velAbs"
  accelAbs/1 -> StopTimeFlow/1(accelRel)  "accelAbs"
  StopTimeFlow/1(tStop) -> tStop/1  "tStop"

== system TosAeb/FcwAebJa_Step_sys/ALG_AEBSystem/ALG_AEBController/AEB_LongTgtInfoCalc/LongObjCalcInfoCalc/AEBS_LongCollisionAssesApp/collisionSeverityHighCalc/collisionSeverityHighCalc1/CollisionAssessHighCalcVersion3/CollisionAssessHighCalc2/CalcBrakePhase1/tObjStopCalc/tStopCalc ==
  accelAbs : Inport
  velAbs : Inport  Port=2
  StopTimeFlow : Stateflow chart  TreatAsAtomicUnit=on
  tStop : Outport
  wires:
  velAbs/1 -> StopTimeFlow/2(velRel)  "velAbs"
  accelAbs/1 -> StopTimeFlow/1(accelRel)  "accelAbs"
  StopTimeFlow/1(tStop) -> tStop/1  "tStop"

== system TosAeb/FcwAebJa_Step_sys/ALG_AEBSystem/ALG_AEBController/AEB_LongTgtInfoCalc/LongObjCalcInfoCalc/AEBS_LongCollisionAssesApp/collisionSeverityHighCalc/collisionSeverityHighCalc1/CollisionAssessHighCalcVersion3/CollisionAssessHighCalc2/CalcBrakePhase1/timeEgovDelataCalc/timeEgovDelata ==
  accelAbs : Inport
  velAbs : Inport  Port=2
  StopTimeFlow : Stateflow chart  TreatAsAtomicUnit=on
  tStop : Outport
  wires:
  velAbs/1 -> StopTimeFlow/2(velRel)  "velAbs"
  accelAbs/1 -> StopTimeFlow/1(accelRel)  "accelAbs"
  StopTimeFlow/1(tStop) -> tStop/1  "tStop"

== system TosAeb/FcwAebJa_Step_sys/ALG_AEBSystem/ALG_AEBController/AEB_LongTgtInfoCalc/LongObjCalcInfoCalc/AEBS_LongCollisionAssesApp/collisionSeverityHighCalc/collisionSeverityHighCalc1/CollisionAssessHighCalcVersion3/CollisionAssessHighCalc2/CalcBrakePhase1/timeEgovDelataCalc/velMaxReudceFiltCalc ==
  v0Ego : Inport
  velMaxReudce : Inport  Port=2
  MinMax : MinMax  Inputs=2
  velMaxReudceFilt : Outport
  wires:
  MinMax/1 -> velMaxReudceFilt/1
  velMaxReudce/1 -> MinMax/2
  v0Ego/1 -> MinMax/1

== system TosAeb/FcwAebJa_Step_sys/ALG_AEBSystem/ALG_AEBController/AEB_LongTgtInfoCalc/LongObjCalcInfoCalc/AEBS_LongCollisionAssesApp/collisionSeverityHighCalc/collisionSeverityHighCalc1/CollisionAssessHighCalcVersion3/CollisionAssessHighCalc2/CalcBrakePhase2/BrkSysRuntimeFhase2/If Action Subsystem ==
  a0Ego : Inport
  accelMaxDec : Inport  Port=2
  accelGradient : Inport  Port=3
  Action Port : ActionPort
  Divide : Product  Inputs=*/
  Subtract : Sum  Inputs=+-
  tTime : Outport
  wires:
  accelMaxDec/1 -> Subtract/1
  a0Ego/1 -> Subtract/2
  Subtract/1 -> Divide/1
  accelGradient/1 -> Divide/2
  Divide/1 -> tTime/1

== system TosAeb/FcwAebJa_Step_sys/ALG_AEBSystem/ALG_AEBController/AEB_LongTgtInfoCalc/LongObjCalcInfoCalc/AEBS_LongCollisionAssesApp/collisionSeverityHighCalc/collisionSeverityHighCalc1/CollisionAssessHighCalcVersion3/CollisionAssessHighCalc2/CalcBrakePhase2/BrkSysRuntimeFhase2/If Action Subsystem1 ==
  Action Port : ActionPort
  Constant : Constant  Value=0
  tTime : Outport
  wires:
  Constant/1 -> tTime/1

== system TosAeb/FcwAebJa_Step_sys/ALG_AEBSystem/ALG_AEBController/AEB_LongTgtInfoCalc/LongObjCalcInfoCalc/AEBS_LongCollisionAssesApp/collisionSeverityHighCalc/collisionSeverityHighCalc1/CollisionAssessHighCalcVersion3/CollisionAssessHighCalc2/CalcBrakePhase2/BrkSysRuntimeFhase2/accelEgoLessAccelmaxReduce ==
  accelMaxDec : Inport
  a0Ego : Inport  Port=2
  Relational Operator : RelationalOperator  Operator=<  OutDataTypeStr=boolean  RndMeth=Simplest
  Flg : Outport
  wires:
  Relational Operator/1 -> Flg/1
  accelMaxDec/1 -> Relational Operator/1
  a0Ego/1 -> Relational Operator/2

== system TosAeb/FcwAebJa_Step_sys/ALG_AEBSystem/ALG_AEBController/AEB_LongTgtInfoCalc/LongObjCalcInfoCalc/AEBS_LongCollisionAssesApp/collisionSeverityHighCalc/collisionSeverityHighCalc1/CollisionAssessHighCalcVersion3/CollisionAssessHighCalc2/CalcBrakePhase2/tEgoStopCalc/tStopCalc ==
  accelAbs : Inport
  velAbs : Inport  Port=2
  StopTimeFlow : Stateflow chart  TreatAsAtomicUnit=on
  tStop : Outport
  wires:
  velAbs/1 -> StopTimeFlow/2(velRel)  "velAbs"
  accelAbs/1 -> StopTimeFlow/1(accelRel)  "accelAbs"
  StopTimeFlow/1(tStop) -> tStop/1  "tStop"

== system TosAeb/FcwAebJa_Step_sys/ALG_AEBSystem/ALG_AEBController/AEB_LongTgtInfoCalc/LongObjCalcInfoCalc/AEBS_LongCollisionAssesApp/collisionSeverityHighCalc/collisionSeverityHighCalc1/CollisionAssessHighCalcVersion3/CollisionAssessHighCalc2/CalcBrakePhase2/tObjStopCalc/tStopCalc ==
  accelAbs : Inport
  velAbs : Inport  Port=2
  StopTimeFlow : Stateflow chart  TreatAsAtomicUnit=on
  tStop : Outport
  wires:
  velAbs/1 -> StopTimeFlow/2(velRel)  "velAbs"
  accelAbs/1 -> StopTimeFlow/1(accelRel)  "accelAbs"
  StopTimeFlow/1(tStop) -> tStop/1  "tStop"

== system TosAeb/FcwAebJa_Step_sys/ALG_AEBSystem/ALG_AEBController/AEB_LongTgtInfoCalc/LongObjCalcInfoCalc/AEBS_LongCollisionAssesApp/collisionSeverityHighCalc/collisionSeverityHighCalc1/CollisionAssessHighCalcVersion3/CollisionAssessHighCalc2/CalcBrakePhase2/timeEgovDelataCalc/timeEgovDelata ==
  accelAbs : Inport
  velAbs : Inport  Port=2
  StopTimeFlow : Stateflow chart  TreatAsAtomicUnit=on
  tStop : Outport
  wires:
  velAbs/1 -> StopTimeFlow/2(velRel)  "velAbs"
  accelAbs/1 -> StopTimeFlow/1(accelRel)  "accelAbs"
  StopTimeFlow/1(tStop) -> tStop/1  "tStop"

== system TosAeb/FcwAebJa_Step_sys/ALG_AEBSystem/ALG_AEBController/AEB_LongTgtInfoCalc/LongObjCalcInfoCalc/AEBS_LongCollisionAssesApp/collisionSeverityHighCalc/collisionSeverityHighCalc1/CollisionAssessHighCalcVersion3/CollisionAssessHighCalc2/CalcBrakePhase2/timeEgovDelataCalc/velMaxReudceFiltCalc ==
  v0Ego : Inport
  velMaxReudce : Inport  Port=2
  MinMax : MinMax  Inputs=2
  velMaxReudceFilt : Outport
  wires:
  MinMax/1 -> velMaxReudceFilt/1
  velMaxReudce/1 -> MinMax/2
  v0Ego/1 -> MinMax/1

== system TosAeb/FcwAebJa_Step_sys/ALG_AEBSystem/ALG_AEBController/AEB_LongTgtInfoCalc/LongObjCalcInfoCalc/AEBS_LongCollisionAssesApp/collisionSeverityHighCalc/collisionSeverityHighCalc1/CollisionAssessHighCalcVersion3/CollisionAssessHighCalc2/CalcBrakePhase3/BrkSysRuntime/Subsystem ==
  a0Obj : Inport
  accelMaxDec : Inport  Port=2
  Abs : Abs
  Compare To Constant : simulink/Logic and Bit Operations/Compare To Constant  relop=<  const=1e-6  OutDataTypeStr=boolean
  Subtract : Sum  Inputs=+-
  Flg : Outport
  wires:
  Abs/1 -> Compare To Constant/1
  accelMaxDec/1 -> Subtract/2
  a0Obj/1 -> Subtract/1
  Subtract/1 -> Abs/1
  Compare To Constant/1 -> Flg/1

== system TosAeb/FcwAebJa_Step_sys/ALG_AEBSystem/ALG_AEBController/AEB_LongTgtInfoCalc/LongObjCalcInfoCalc/AEBS_LongCollisionAssesApp/collisionSeverityHighCalc/collisionSeverityHighCalc1/CollisionAssessHighCalcVersion3/CollisionAssessHighCalc2/CalcBrakePhase3/BrkSysRuntime/a0ObjGreatSituation ==
  vtEgo : Inport
  accelMaxDec : Inport  Port=2
  v0Obj : Inport  Port=3
  a0Obj : Inport  Port=4
  Action Port : ActionPort
  If : If  IfExpression=u1
  Merge : Merge
  Subsystem : Subsystem
  vtEgoGreatSituation : Subsystem  TreatAsAtomicUnit=on
  vtEgoLessSituation : Subsystem  TreatAsAtomicUnit=on
  tTime : Outport
  wires:
  [vtEgoGreat] -> vtEgoGreatSituation/Action
  If/2 -> [vtEgoGreat]
  Subsystem/1(Flg) -> If/1
  vtEgo/1 -> vtEgoLessSituation/2(vtEgo), Subsystem/1(vtEgo)
  v0Obj/1 -> vtEgoLessSituation/1(v0Obj), Subsystem/2(v0Obj)
  If/1 -> vtEgoLessSituation/Action
  vtEgoLessSituation/1(tTime) -> Merge/1
  vtEgoGreatSituation/1(tTime) -> Merge/2
  Merge/1 -> tTime/1
  accelMaxDec/1 -> vtEgoLessSituation/3(accelMaxDec)
  a0Obj/1 -> vtEgoLessSituation/4(a0Obj)

== system TosAeb/FcwAebJa_Step_sys/ALG_AEBSystem/ALG_AEBController/AEB_LongTgtInfoCalc/LongObjCalcInfoCalc/AEBS_LongCollisionAssesApp/collisionSeverityHighCalc/collisionSeverityHighCalc1/CollisionAssessHighCalcVersion3/CollisionAssessHighCalc2/CalcBrakePhase3/BrkSysRuntime/a0ObjLessSituation ==
  vtEgo : Inport
  accelMaxDec : Inport  Port=2
  Action Port : ActionPort
  Divide : Product  Inputs=*/
  Unary Minus : UnaryMinus
  tTime : Outport
  wires:
  vtEgo/1 -> Unary Minus/1
  Unary Minus/1 -> Divide/1
  accelMaxDec/1 -> Divide/2
  Divide/1 -> tTime/1

== system TosAeb/FcwAebJa_Step_sys/ALG_AEBSystem/ALG_AEBController/AEB_LongTgtInfoCalc/LongObjCalcInfoCalc/AEBS_LongCollisionAssesApp/collisionSeverityHighCalc/collisionSeverityHighCalc1/CollisionAssessHighCalcVersion3/CollisionAssessHighCalc2/CalcBrakePhase3/tEgoStopCalc/tStopCalc ==
  accelAbs : Inport
  velAbs : Inport  Port=2
  StopTimeFlow : Stateflow chart  TreatAsAtomicUnit=on
  tStop : Outport
  wires:
  velAbs/1 -> StopTimeFlow/2(velRel)  "velAbs"
  accelAbs/1 -> StopTimeFlow/1(accelRel)  "accelAbs"
  StopTimeFlow/1(tStop) -> tStop/1  "tStop"

== system TosAeb/FcwAebJa_Step_sys/ALG_AEBSystem/ALG_AEBController/AEB_LongTgtInfoCalc/LongObjCalcInfoCalc/AEBS_LongCollisionAssesApp/collisionSeverityHighCalc/collisionSeverityHighCalc1/CollisionAssessHighCalcVersion3/CollisionAssessHighCalc2/CalcBrakePhase3/tObjStopCalc/tStopCalc ==
  accelAbs : Inport
  velAbs : Inport  Port=2
  StopTimeFlow : Stateflow chart  TreatAsAtomicUnit=on
  tStop : Outport
  wires:
  velAbs/1 -> StopTimeFlow/2(velRel)  "velAbs"
  accelAbs/1 -> StopTimeFlow/1(accelRel)  "accelAbs"
  StopTimeFlow/1(tStop) -> tStop/1  "tStop"

== system TosAeb/FcwAebJa_Step_sys/ALG_AEBSystem/ALG_AEBController/AEB_LongTgtInfoCalc/LongObjCalcInfoCalc/AEBS_LongCollisionAssesApp/collisionSeverityHighCalc/collisionSeverityHighCalc1/CollisionAssessHighCalcVersion3/CollisionAssessHighCalc2/CalcBrakePhase3/timeEgovDelataCalc/timeEgovDelata ==
  accelAbs : Inport
  velAbs : Inport  Port=2
  StopTimeFlow : Stateflow chart  TreatAsAtomicUnit=on
  tStop : Outport
  wires:
  velAbs/1 -> StopTimeFlow/2(velRel)  "velAbs"
  accelAbs/1 -> StopTimeFlow/1(accelRel)  "accelAbs"
  StopTimeFlow/1(tStop) -> tStop/1  "tStop"

== system TosAeb/FcwAebJa_Step_sys/ALG_AEBSystem/ALG_AEBController/AEB_LongTgtInfoCalc/LongObjCalcInfoCalc/AEBS_LongCollisionAssesApp/collisionSeverityHighCalc/collisionSeverityHighCalc1/CollisionAssessHighCalcVersion3/CollisionAssessHighCalc2/CalcBrakePhase3/timeEgovDelataCalc/velMaxReudceFiltCalc ==
  v0Ego : Inport
  velMaxReudce : Inport  Port=2
  MinMax : MinMax  Inputs=2
  velMaxReudceFilt : Outport
  wires:
  MinMax/1 -> velMaxReudceFilt/1
  velMaxReudce/1 -> MinMax/2
  v0Ego/1 -> MinMax/1

== system TosAeb/AEB_Objsel/TA_Processor/AEB_ObjSelect/TargetInfoCheck/Data_precess/TA_EffectCalculate/Obj_FilterFlgCalculate/Long_ObjFilter/OverlapCalculate/BoudingBoxCalculate_Else/Overlap_FlgCalculate/AbsPosn_InsectionFlg/ObjBoundingBox/ObjLLCoordinate ==
  inputSignals : Inport
  Add : Sum  Inputs=+-  OutDataTypeStr=single
  Add1 : Sum  OutDataTypeStr=single
  Bus Selector : BusSelector  OutputSignals=dxPreRefPointObj,ObjWidth,sincosCoef.sinCoef
  Bus Selector1 : BusSelector  OutputSignals=dyPreRefPointObj,ObjWidth,sincosCoef.cosCoef
  Gain : Gain  Gain=0.5  OutDataTypeStr=single
  Gain1 : Gain  Gain=0.5  OutDataTypeStr=single
  Product1 : Product  OutDataTypeStr=single
  Product3 : Product  OutDataTypeStr=single
  dxPre_LLObj : Outport
  dyPreLLObj : Outport  Port=2
  wires:
  Bus Selector1/1 -> Add1/1  "<dyPreRefPointObj>"
  Bus Selector1/3 -> Product3/2  "<cosCoef>"
  Bus Selector1/2 -> Product3/1  "<ObjWidth>"
  Product3/1 -> Gain/1
  Add1/1 -> dyPreLLObj/1
  Add/1 -> dxPre_LLObj/1
  Bus Selector/1 -> Add/1  "<dxPreRefPointObj>"
  Product1/1 -> Gain1/1
  Bus Selector/3 -> Product1/2  "<sinCoef>"
  Bus Selector/2 -> Product1/1  "<ObjWidth>"
  inputSignals/1 -> Bus Selector1/1, Bus Selector/1
  Gain/1 -> Add1/2
  Gain1/1 -> Add/2

== system TosAeb/AEB_Objsel/TA_Processor/AEB_ObjSelect/TargetInfoCheck/Data_precess/TA_EffectCalculate/Obj_FilterFlgCalculate/Long_ObjFilter/OverlapCalculate/BoudingBoxCalculate_Else/Overlap_FlgCalculate/AbsPosn_InsectionFlg/ObjBoundingBox/ObjLUCoordinate ==
  inputSignals : Inport
  Add : Sum  Inputs=++-  OutDataTypeStr=single
  Add1 : Sum  Inputs=+++  OutDataTypeStr=single
  Bus Selector : BusSelector  OutputSignals=dxPreRefPointObj,ObjLength,sincosCoef.cosCoef,ObjWidth,sincosCoef.sinCoef
  Bus Selector1 : BusSelector  OutputSignals=dyPreRefPointObj,ObjLength,sincosCoef.sinCoef,ObjWidth,sincosCoef.cosCoef
  Gain : Gain  Gain=0.5  OutDataTypeStr=single
  Gain1 : Gain  Gain=0.5  OutDataTypeStr=single
  Product{,1..3} (4 alike) : Product  OutDataTypeStr=single
  dxPreLUObj : Outport
  dyPreLUobj : Outport  Port=2
  wires:
  inputSignals/1 -> Bus Selector1/1, Bus Selector/1
  Bus Selector/2 -> Product/1  "<ObjLength>"
  Bus Selector/3 -> Product/2  "<cosCoef>"
  Bus Selector/4 -> Product1/1  "<ObjWidth>"
  Bus Selector/5 -> Product1/2  "<sinCoef>"
  Product1/1 -> Gain1/1
  Bus Selector/1 -> Add/1  "<dxPreRefPointObj>"
  Product/1 -> Add/2
  Add/1 -> dxPreLUObj/1
  Add1/1 -> dyPreLUobj/1
  Product2/1 -> Add1/2
  Product3/1 -> Gain/1
  Bus Selector1/2 -> Product2/1  "<ObjLength>"
  Bus Selector1/4 -> Product3/1  "<ObjWidth>"
  Bus Selector1/3 -> Product2/2  "<sinCoef>"
  Bus Selector1/5 -> Product3/2  "<cosCoef>"
  Bus Selector1/1 -> Add1/1  "<dyPreRefPointObj>"
  Gain/1 -> Add1/3
  Gain1/1 -> Add/3

== system TosAeb/AEB_Objsel/TA_Processor/AEB_ObjSelect/TargetInfoCheck/Data_precess/TA_EffectCalculate/Obj_FilterFlgCalculate/Long_ObjFilter/OverlapCalculate/BoudingBoxCalculate_Else/Overlap_FlgCalculate/AbsPosn_InsectionFlg/ObjBoundingBox/ObjRLCoordinate ==
  inputSignals : Inport
  Add : Sum  OutDataTypeStr=single
  Add1 : Sum  Inputs=+-  OutDataTypeStr=single
  Bus Selector : BusSelector  OutputSignals=dxPreRefPointObj,ObjWidth,sincosCoef.sinCoef
  Bus Selector1 : BusSelector  OutputSignals=dyPreRefPointObj,ObjWidth,sincosCoef.cosCoef
  Gain : Gain  Gain=0.5  OutDataTypeStr=single
  Gain1 : Gain  Gain=0.5  OutDataTypeStr=single
  Product1 : Product  OutDataTypeStr=single
  Product3 : Product  OutDataTypeStr=single
  dxPreRLObj : Outport
  dyPreRLObj : Outport  Port=2
  wires:
  Bus Selector1/1 -> Add1/1  "<dyPreRefPointObj>"
  Bus Selector1/3 -> Product3/2  "<cosCoef>"
  Bus Selector1/2 -> Product3/1  "<ObjWidth>"
  Product3/1 -> Gain1/1
  Add1/1 -> dyPreRLObj/1
  Add/1 -> dxPreRLObj/1
  Bus Selector/1 -> Add/1  "<dxPreRefPointObj>"
  Product1/1 -> Gain/1
  Bus Selector/3 -> Product1/2  "<sinCoef>"
  Bus Selector/2 -> Product1/1  "<ObjWidth>"
  inputSignals/1 -> Bus Selector1/1, Bus Selector/1
  Gain/1 -> Add/2
  Gain1/1 -> Add1/2

== system TosAeb/AEB_Objsel/TA_Processor/AEB_ObjSelect/TargetInfoCheck/Data_precess/TA_EffectCalculate/Obj_FilterFlgCalculate/Long_ObjFilter/OverlapCalculate/BoudingBoxCalculate_Else/Overlap_FlgCalculate/AbsPosn_InsectionFlg/ObjBoundingBox/ObjRUCoordinate ==
  inputSignals : Inport
  Add : Sum  Inputs=+++  OutDataTypeStr=single
  Add1 : Sum  Inputs=++-  OutDataTypeStr=single
  Bus Selector : BusSelector  OutputSignals=dxPreRefPointObj,ObjLength,sincosCoef.cosCoef,ObjWidth,sincosCoef.sinCoef
  Bus Selector1 : BusSelector  OutputSignals=dyPreRefPointObj,ObjLength,sincosCoef.sinCoef,ObjWidth,sincosCoef.cosCoef
  Gain : Gain  Gain=0.5  OutDataTypeStr=single
  Gain1 : Gain  Gain=0.5  OutDataTypeStr=single
  Product{,1..3} (4 alike) : Product  OutDataTypeStr=single
  dxPreRUObj : Outport
  dyPreRUObj : Outport  Port=2
  wires:
  inputSignals/1 -> Bus Selector1/1, Bus Selector/1
  Bus Selector/2 -> Product/1  "<ObjLength>"
  Bus Selector/3 -> Product/2  "<cosCoef>"
  Bus Selector/4 -> Product1/1  "<ObjWidth>"
  Bus Selector/5 -> Product1/2  "<sinCoef>"
  Product1/1 -> Gain1/1
  Bus Selector/1 -> Add/1  "<dxPreRefPointObj>"
  Product/1 -> Add/2
  Add/1 -> dxPreRUObj/1
  Add1/1 -> dyPreRUObj/1
  Product2/1 -> Add1/2
  Product3/1 -> Gain/1
  Bus Selector1/2 -> Product2/1  "<ObjLength>"
  Bus Selector1/4 -> Product3/1  "<ObjWidth>"
  Bus Selector1/3 -> Product2/2  "<sinCoef>"
  Bus Selector1/5 -> Product3/2  "<cosCoef>"
  Bus Selector1/1 -> Add1/1  "<dyPreRefPointObj>"
  Gain/1 -> Add1/3
  Gain1/1 -> Add/3

== system TosAeb/AEB_Objsel/TA_Processor/AEB_ObjSelect/TargetInfoCheck/Data_precess/TA_EffectCalculate/Obj_FilterFlgCalculate/Long_ObjFilter/OverlapCalculate/BoudingBoxCalculate_Else/Overlap_FlgCalculate/AbsPosn_InsectionFlg/SubjBoundingBox/SubjLLCoordinate ==
  inputSignals : Inport
  Add2 : Sum  Inputs=+---  OutDataTypeStr=single
  Add3 : Sum  Inputs=+-+-  OutDataTypeStr=single
  Bus Selector2 : BusSelector  OutputSignals=dxPreRefPoint,dxRearBumperToRearAxis,sincosCoef.cosCoef,subjWidth,sincosCoef.sinCoef,Long_Offset
  Bus Selector3 : BusSelector  OutputSignals=dyPreRefPoint,dxRearBumperToRearAxis,sincosCoef.sinCoef,subjWidth,sincosCoef.cosCoef,Lat_Offset
  Gain2 : Gain  Gain=0.5  OutDataTypeStr=single
  Gain3 : Gain  Gain=0.5  OutDataTypeStr=single
  Product{4..7} (4 alike) : Product  OutDataTypeStr=single
  dxPreLL : Outport
  dyPreLL : Outport  Port=2
  wires:
  Gain3/1 -> Add3/3
  Bus Selector3/3 -> Product6/2  "<sinCoef>"
  Add3/1 -> dyPreLL/1
  Product7/1 -> Gain3/1
  Product6/1 -> Add3/2
  Product4/1 -> Add2/2
  Bus Selector3/5 -> Product7/2  "<cosCoef>"
  Product5/1 -> Gain2/1
  Gain2/1 -> Add2/3
  Add2/1 -> dxPreLL/1
  Bus Selector3/4 -> Product7/1  "<subjWidth>"
  Bus Selector3/2 -> Product6/1  "<dxRearBumperToRearAxis>"
  Bus Selector3/1 -> Add3/1  "<dyPreRefPoint>"
  inputSignals/1 -> Bus Selector3/1, Bus Selector2/1
  Bus Selector2/4 -> Product5/1  "<subjWidth>"
  Bus Selector2/2 -> Product4/1  "<dxRearBumperToRearAxis>"
  Bus Selector2/3 -> Product4/2  "<cosCoef>"
  Bus Selector2/1 -> Add2/1  "<dxPreRefPoint>"
  Bus Selector2/5 -> Product5/2  "<sinCoef>"
  Bus Selector2/6 -> Add2/4  "<Long_Offset>"
  Bus Selector3/6 -> Add3/4  "<Lat_Offset>"
